simulink model "CalPrmAndSigMgrForCmptmtTCtrl"  (R2022b)
settings: Solver=FixedStepDiscrete  StartTime=0.0  StopTime=10.0  FixedStep=1  RelTol=1e-3
settings Model: Creator=blue.ge  LastModifiedBy=blue.ge
settings DataIOCC: SaveFormat=StructureWithTime
settings OptimizationCC: DefaultParameterBehavior=Inlined  EnhancedBackFolding=on  PassReuseOutputArgsAs=Individual arguments  ZeroExternalMemoryAtStartup=off  ZeroInternalMemoryAtStartup=off  OptimizeBlockOrder=speed  DifferentSizesBufferReuse=on  OptimizationCustomize=off  EfficientTunableParamExpr=on
settings DebuggingCC: ParameterTunabilityLossMsg=error
settings ModelReferenceCC: ModelReferenceNumInstancesAllowed=Single
settings SFSimCC: MATLABDynamicMemAlloc=off
settings RTWCC: Description=AUTOSAR  SystemTargetFile=autosar.tlc  GenCodeOnly=on  TemplateMakefile=ert_default_tmf
settings CodeAppCC: ForceParamTrailComments=on  IgnoreCustomStorageClasses=off  OperatorAnnotations=on  SimulinkDataObjDesc=on  SFDataObjDesc=on  InsertBlockDesc=on
settings STFCustomTargetCC: TargetLangStandard=C99 (ISO)  MultiwordLength=256  ParMdlRefBuildCompliant=on  CompOptLevelCompliant=on  IncludeMdlTerminateFcn=off  CombineOutputUpdateFcns=on  SuppressErrorStatus=on  ERTCustomFileBanners=on  SupportNonFinite=off  SupportComplex=off  SupportContinuousTime=off  SupportNonInlinedSFcns=off  RemoveResetFunc=on  MATLABClassNameForMDSCustomization=Simulink.SoftwareTarget.ERTCustomization  AutosarCompliant=on  UseToolchainInfoCompliant=on  LookupTableObjectStructAxisOrder=2,1,3,4,...  LUTObjectStructOrderEvenSpacing=Size,Table,Breakpoints  SystemTargetFile=autosar.tlc
settings ERTTargetCC: TargetLangStandard=C99 (ISO)  ConcurrentExecutionCompliant=off  IncludeMdlTerminateFcn=off  SuppressErrorStatus=on  SupportNonFinite=off  SupportComplex=off  ModelStepFunctionPrototypeControlCompliant=off  CPPClassGenCompliant=off  AutosarCompliant=on  LookupTableObjectStructAxisOrder=2,1,3,4,...  LUTObjectStructOrderEvenSpacing=Size,Table,Breakpoints  InlinedParameterPlacement=NonHierarchical  ERTCustomFileTemplate=example_file_process.tlc

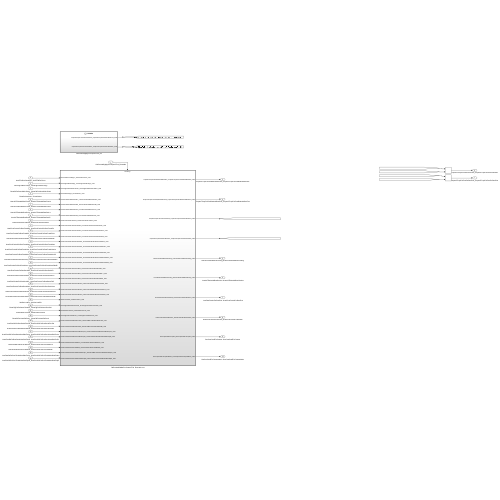
[thumbnail: Simulink's stored preview of the top-level diagram]
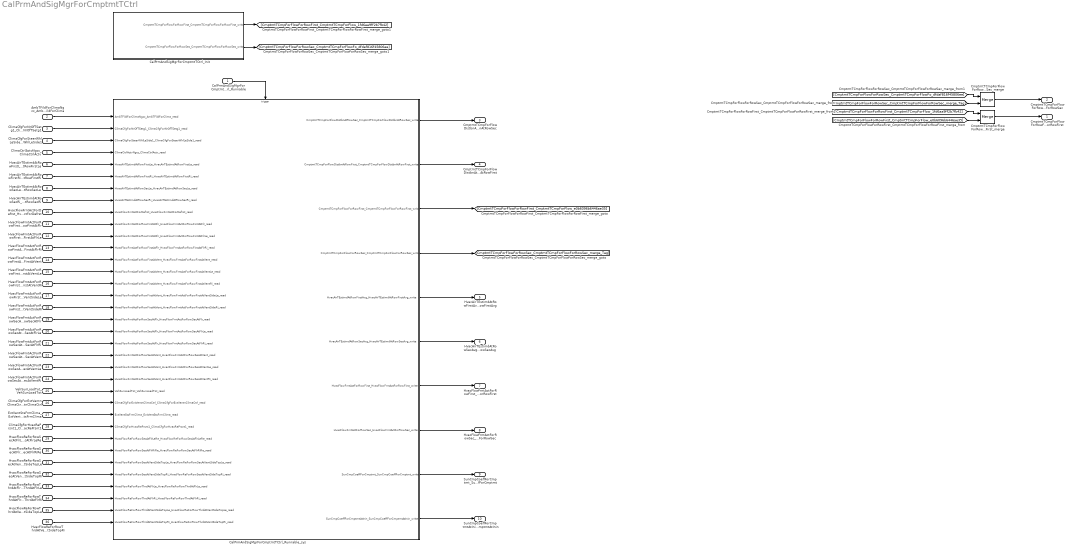
== system CalPrmAndSigMgrForCmptmtTCtrl ==
  CalPrmAndSigMgrForCmptmtTCtrl_Runnable : Inport  SampleTime=1  OutputFunctionCall=on
  AmbTFildForClimaNgcc_AmbTFildForClima : Inport  Port=2  OutDataTypeStr=T30  PortDimensions=1
  ClimaCfgForNrOfTSetg1_ClimaCfgForNrOfTSetg1 : Inport  Port=3  OutDataTypeStr=Enum: ClimaCfgForNrOfTSetg1  PortDimensions=1
  ClimaCfgForSteerWhlLeSide1_ClimaCfgForSteerWhlLeSide1 : Inport  Port=4  OutDataTypeStr=Boolean  PortDimensions=1
  ClimaCtrlActvNgcc_ClimaCtrlActv : Inport  Port=5  OutDataTypeStr=Boolean  PortDimensions=1
  HvacAirTEstimdAtRowFirstLe_HvacAirTEstimdAtRowFirstLe : Inport  Port=6  OutDataTypeStr=T30  PortDimensions=1
  HvacAirTEstimdAtRowFirstRi_HvacAirTEstimdAtRowFirstRi : Inport  Port=7  OutDataTypeStr=T30  PortDimensions=1
  HvacAirTEstimdAtRowSecLe_HvacAirTEstimdAtRowSecLe : Inport  Port=8  OutDataTypeStr=T30  PortDimensions=1
  HvacAirTEstimdAtRowSecRi_HvacAirTEstimdAtRowSecRi : Inport  Port=9  OutDataTypeStr=T30  PortDimensions=1
  HvacFlowFrntActForDefrst_HvacFlowFrntActForDefrst : Inport  Port=10  OutDataTypeStr=AirFlow30  PortDimensions=1
  HvacFlowFrntActForRowFirstAtFlr_HvacFlowFrntActForRowFirstAtFlr : Inport  Port=11  OutDataTypeStr=AirFlow30  PortDimensions=1
  HvacFlowFrntActForRowFirstAtFlr_HvacFlowFrntActForRowFirstAtFlrLe : Inport  Port=12  OutDataTypeStr=AirFlow30  PortDimensions=1
  HvacFlowFrntActForRowFirstAtFlr_HvacFlowFrntActForRowFirstAtFlrRi : Inport  Port=13  OutDataTypeStr=AirFlow30  PortDimensions=1
  HvacFlowFrntActForRowFirstAtVent_HvacFlowFrntActForRowFirstAtVent : Inport  Port=14  OutDataTypeStr=AirFlow30  PortDimensions=1
  HvacFlowFrntActForRowFirstAtVent_HvacFlowFrntActForRowFirstAtVentLe : Inport  Port=15  OutDataTypeStr=AirFlow30  PortDimensions=1
  HvacFlowFrntActForRowFirstAtVent_HvacFlowFrntActForRowFirstAtVentRi : Inport  Port=16  OutDataTypeStr=AirFlow30  PortDimensions=1
  HvacFlowFrntActForRowFirstAtVent_HvacFlowFrntActForRowFirstAtVentSideLe : Inport  Port=17  OutDataTypeStr=AirFlow30  PortDimensions=1
  HvacFlowFrntActForRowFirstAtVent_HvacFlowFrntActForRowFirstAtVentSideRi : Inport  Port=18  OutDataTypeStr=AirFlow30  PortDimensions=1
  HvacFlowFrntActForRowSecAtFlr_HvacFlowFrntActForRowSecAtFlr : Inport  Port=19  OutDataTypeStr=AirFlow30  PortDimensions=1
  HvacFlowFrntActForRowSecAtFlr_HvacFlowFrntActForRowSecAtFlrLe : Inport  Port=20  OutDataTypeStr=AirFlow30  PortDimensions=1
  HvacFlowFrntActForRowSecAtFlr_HvacFlowFrntActForRowSecAtFlrRi : Inport  Port=21  OutDataTypeStr=AirFlow30  PortDimensions=1
  HvacFlowFrntActForRowSecAtVent_HvacFlowFrntActForRowSecAtVent : Inport  Port=22  OutDataTypeStr=AirFlow30  PortDimensions=1
  HvacFlowFrntActForRowSecAtVent_HvacFlowFrntActForRowSecAtVentLe : Inport  Port=23  OutDataTypeStr=AirFlow30  PortDimensions=1
  HvacFlowFrntActForRowSecAtVent_HvacFlowFrntActForRowSecAtVentRi : Inport  Port=24  OutDataTypeStr=AirFlow30  PortDimensions=1
  VehSunLoadTot_VehSunLoadTot : Inport  Port=25  OutDataTypeStr=SunLoad30  PortDimensions=1
  ClimaCfgForExtVentnClimaCtrl_ClimaCfgForExtVentnClimaCtrl : Inport  Port=26  OutDataTypeStr=Enum: CarCfgForExtVentnClimaCtrl  PortDimensions=1
  ExtVentStsFrmClima_ExtVentStsFrmClima : Inport  Port=27  OutDataTypeStr=Enum: OnOff1  PortDimensions=1
  ClimaCfgForHvacRePrsnt1_ClimaCfgForHvacRePrsnt1 : Inport  Port=28  OutDataTypeStr=Enum: CarCfgForHvacRe  PortDimensions=1
  HvacFlowReForRowSecAtFlrLeRe_HvacFlowReForRowSecAtFlrLeRe : Inport  Port=29  OutDataTypeStr=AirFlow30  PortDimensions=1
  HvacFlowReForRowSecAtFlrRiRe_HvacFlowReForRowSecAtFlrRiRe : Inport  Port=30  OutDataTypeStr=AirFlow30  PortDimensions=1
  HvacFlowReForRowSecAtVentSideTopLe_HvacFlowReForRowSecAtVentSideTopLe : Inport  Port=31  OutDataTypeStr=AirFlow30  PortDimensions=1
  HvacFlowReForRowSecAtVentSideTopRi_HvacFlowReForRowSecAtVentSideTopRi : Inport  Port=32  OutDataTypeStr=AirFlow30  PortDimensions=1
  HvacFlowReForRowThrdAtFlrLe_HvacFlowReForRowThrdAtFlrLe : Inport  Port=33  OutDataTypeStr=AirFlow30  PortDimensions=1
  HvacFlowReForRowThrdAtFlrRi_HvacFlowReForRowThrdAtFlrRi : Inport  Port=34  OutDataTypeStr=AirFlow30  PortDimensions=1
  HvacFlowReForRowThrdAtVentSideTopLe_HvacFlowReForRowThrdAtVentSideTopLe : Inport  Port=35  OutDataTypeStr=AirFlow30  PortDimensions=1
  HvacFlowReForRowThrdAtVentSideTopRi_HvacFlowReForRowThrdAtVentSideTopRi : Inport  Port=36  OutDataTypeStr=AirFlow30  PortDimensions=1
  CalPrmAndSigMgrForCmptmtTCtrl_Init : Subsystem
  CalPrmAndSigMgrForCmptmtTCtrl_Runnable_sys : Subsystem
  CmptmtTCmpForFlowForRowFirst_CmptmtTCmpForFlowForRowFirst_merge : Merge
  CmptmtTCmpForFlowForRowSec_CmptmtTCmpForFlowForRowSec_merge : Merge
  CmptmtTCmpForFlowForRowFirst_CmptmtTCmpForFlowForRowFirst : Outport  OutDataTypeStr=Fac30  PortDimensions=1
  CmptmtTCmpForFlowForRowSec_CmptmtTCmpForFlowForRowSec : Outport  Port=2  OutDataTypeStr=Fac30  PortDimensions=1
  CmptmtTCmpForFlowDistbnAtRowSec_CmptmtTCmpForFlowDistbnAtRowSec : Outport  Port=3  OutDataTypeStr=Fac30  PortDimensions=1
  CmptmtTCmpForFlowDistbnAtRowFirst_CmptmtTCmpForFlowDistbnAtRowFirst : Outport  Port=4  OutDataTypeStr=Fac30  PortDimensions=1
  HvacAirTEstimdAtRowFirstAvg_HvacAirTEstimdAtRowFirstAvg : Outport  Port=5  OutDataTypeStr=T30  PortDimensions=1
  HvacAirTEstimdAtRowSecAvg_HvacAirTEstimdAtRowSecAvg : Outport  Port=6  OutDataTypeStr=T30  PortDimensions=1
  HvacFlowFrntActForRowFirst_HvacFlowFrntActForRowFirst : Outport  Port=7  OutDataTypeStr=AirFlow30  PortDimensions=1
  HvacFlowFrntActForRowSec_HvacFlowFrntActForRowSec : Outport  Port=8  OutDataTypeStr=AirFlow30  PortDimensions=1
  SunCmpCoeffForCmptmt_SunCmpCoeffForCmptmt : Outport  Port=9  OutDataTypeStr=Fac30  PortDimensions=1
  SunCmpCoeffForCmptmtAtInin_SunCmpCoeffForCmptmtAtInin : Outport  Port=10  OutDataTypeStr=FacForSunCmpInin  PortDimensions=1
  wires:
  AmbTFildForClimaNgcc_AmbTFildForClima/1 -> CalPrmAndSigMgrForCmptmtTCtrl_Runnable_sys/1(AmbTFildForClimaNgcc_AmbTFildForClima_read)
  ClimaCfgForNrOfTSetg1_ClimaCfgForNrOfTSetg1/1 -> CalPrmAndSigMgrForCmptmtTCtrl_Runnable_sys/2(ClimaCfgForNrOfTSetg1_ClimaCfgForNrOfTSetg1_read)
  ClimaCfgForSteerWhlLeSide1_ClimaCfgForSteerWhlLeSide1/1 -> CalPrmAndSigMgrForCmptmtTCtrl_Runnable_sys/3(ClimaCfgForSteerWhlLeSide1_ClimaCfgForSteerWhlLeSide1_read)
  ClimaCtrlActvNgcc_ClimaCtrlActv/1 -> CalPrmAndSigMgrForCmptmtTCtrl_Runnable_sys/4(ClimaCtrlActvNgcc_ClimaCtrlActv_read)
  HvacAirTEstimdAtRowFirstLe_HvacAirTEstimdAtRowFirstLe/1 -> CalPrmAndSigMgrForCmptmtTCtrl_Runnable_sys/5(HvacAirTEstimdAtRowFirstLe_HvacAirTEstimdAtRowFirstLe_read)
  HvacAirTEstimdAtRowFirstRi_HvacAirTEstimdAtRowFirstRi/1 -> CalPrmAndSigMgrForCmptmtTCtrl_Runnable_sys/6(HvacAirTEstimdAtRowFirstRi_HvacAirTEstimdAtRowFirstRi_read)
  HvacAirTEstimdAtRowSecLe_HvacAirTEstimdAtRowSecLe/1 -> CalPrmAndSigMgrForCmptmtTCtrl_Runnable_sys/7(HvacAirTEstimdAtRowSecLe_HvacAirTEstimdAtRowSecLe_read)
  HvacAirTEstimdAtRowSecRi_HvacAirTEstimdAtRowSecRi/1 -> CalPrmAndSigMgrForCmptmtTCtrl_Runnable_sys/8(HvacAirTEstimdAtRowSecRi_HvacAirTEstimdAtRowSecRi_read)
  HvacFlowFrntActForDefrst_HvacFlowFrntActForDefrst/1 -> CalPrmAndSigMgrForCmptmtTCtrl_Runnable_sys/9(HvacFlowFrntActForDefrst_HvacFlowFrntActForDefrst_read)
  HvacFlowFrntActForRowFirstAtFlr_HvacFlowFrntActForRowFirstAtFlr/1 -> CalPrmAndSigMgrForCmptmtTCtrl_Runnable_sys/10(HvacFlowFrntActForRowFirstAtFlr_HvacFlowFrntActForRowFirstAtFlr_read)
  HvacFlowFrntActForRowFirstAtFlr_HvacFlowFrntActForRowFirstAtFlrLe/1 -> CalPrmAndSigMgrForCmptmtTCtrl_Runnable_sys/11(HvacFlowFrntActForRowFirstAtFlr_HvacFlowFrntActForRowFirstAtFlrLe_read)
  HvacFlowFrntActForRowFirstAtFlr_HvacFlowFrntActForRowFirstAtFlrRi/1 -> CalPrmAndSigMgrForCmptmtTCtrl_Runnable_sys/12(HvacFlowFrntActForRowFirstAtFlr_HvacFlowFrntActForRowFirstAtFlrRi_read)
  HvacFlowFrntActForRowFirstAtVent_HvacFlowFrntActForRowFirstAtVent/1 -> CalPrmAndSigMgrForCmptmtTCtrl_Runnable_sys/13(HvacFlowFrntActForRowFirstAtVent_HvacFlowFrntActForRowFirstAtVent_read)
  HvacFlowFrntActForRowFirstAtVent_HvacFlowFrntActForRowFirstAtVentLe/1 -> CalPrmAndSigMgrForCmptmtTCtrl_Runnable_sys/14(HvacFlowFrntActForRowFirstAtVent_HvacFlowFrntActForRowFirstAtVentLe_read)
  HvacFlowFrntActForRowFirstAtVent_HvacFlowFrntActForRowFirstAtVentRi/1 -> CalPrmAndSigMgrForCmptmtTCtrl_Runnable_sys/15(HvacFlowFrntActForRowFirstAtVent_HvacFlowFrntActForRowFirstAtVentRi_read)
  HvacFlowFrntActForRowFirstAtVent_HvacFlowFrntActForRowFirstAtVentSideLe/1 -> CalPrmAndSigMgrForCmptmtTCtrl_Runnable_sys/16(HvacFlowFrntActForRowFirstAtVent_HvacFlowFrntActForRowFirstAtVentSideLe_read)
  HvacFlowFrntActForRowFirstAtVent_HvacFlowFrntActForRowFirstAtVentSideRi/1 -> CalPrmAndSigMgrForCmptmtTCtrl_Runnable_sys/17(HvacFlowFrntActForRowFirstAtVent_HvacFlowFrntActForRowFirstAtVentSideRi_read)
  HvacFlowFrntActForRowSecAtFlr_HvacFlowFrntActForRowSecAtFlr/1 -> CalPrmAndSigMgrForCmptmtTCtrl_Runnable_sys/18(HvacFlowFrntActForRowSecAtFlr_HvacFlowFrntActForRowSecAtFlr_read)
  HvacFlowFrntActForRowSecAtFlr_HvacFlowFrntActForRowSecAtFlrLe/1 -> CalPrmAndSigMgrForCmptmtTCtrl_Runnable_sys/19(HvacFlowFrntActForRowSecAtFlr_HvacFlowFrntActForRowSecAtFlrLe_read)
  HvacFlowFrntActForRowSecAtFlr_HvacFlowFrntActForRowSecAtFlrRi/1 -> CalPrmAndSigMgrForCmptmtTCtrl_Runnable_sys/20(HvacFlowFrntActForRowSecAtFlr_HvacFlowFrntActForRowSecAtFlrRi_read)
  HvacFlowFrntActForRowSecAtVent_HvacFlowFrntActForRowSecAtVent/1 -> CalPrmAndSigMgrForCmptmtTCtrl_Runnable_sys/21(HvacFlowFrntActForRowSecAtVent_HvacFlowFrntActForRowSecAtVent_read)
  HvacFlowFrntActForRowSecAtVent_HvacFlowFrntActForRowSecAtVentLe/1 -> CalPrmAndSigMgrForCmptmtTCtrl_Runnable_sys/22(HvacFlowFrntActForRowSecAtVent_HvacFlowFrntActForRowSecAtVentLe_read)
  HvacFlowFrntActForRowSecAtVent_HvacFlowFrntActForRowSecAtVentRi/1 -> CalPrmAndSigMgrForCmptmtTCtrl_Runnable_sys/23(HvacFlowFrntActForRowSecAtVent_HvacFlowFrntActForRowSecAtVentRi_read)
  VehSunLoadTot_VehSunLoadTot/1 -> CalPrmAndSigMgrForCmptmtTCtrl_Runnable_sys/24(VehSunLoadTot_VehSunLoadTot_read)
  ClimaCfgForExtVentnClimaCtrl_ClimaCfgForExtVentnClimaCtrl/1 -> CalPrmAndSigMgrForCmptmtTCtrl_Runnable_sys/25(ClimaCfgForExtVentnClimaCtrl_ClimaCfgForExtVentnClimaCtrl_read)
  ExtVentStsFrmClima_ExtVentStsFrmClima/1 -> CalPrmAndSigMgrForCmptmtTCtrl_Runnable_sys/26(ExtVentStsFrmClima_ExtVentStsFrmClima_read)
  ClimaCfgForHvacRePrsnt1_ClimaCfgForHvacRePrsnt1/1 -> CalPrmAndSigMgrForCmptmtTCtrl_Runnable_sys/27(ClimaCfgForHvacRePrsnt1_ClimaCfgForHvacRePrsnt1_read)
  HvacFlowReForRowSecAtFlrLeRe_HvacFlowReForRowSecAtFlrLeRe/1 -> CalPrmAndSigMgrForCmptmtTCtrl_Runnable_sys/28(HvacFlowReForRowSecAtFlrLeRe_HvacFlowReForRowSecAtFlrLeRe_read)
  HvacFlowReForRowSecAtFlrRiRe_HvacFlowReForRowSecAtFlrRiRe/1 -> CalPrmAndSigMgrForCmptmtTCtrl_Runnable_sys/29(HvacFlowReForRowSecAtFlrRiRe_HvacFlowReForRowSecAtFlrRiRe_read)
  HvacFlowReForRowSecAtVentSideTopLe_HvacFlowReForRowSecAtVentSideTopLe/1 -> CalPrmAndSigMgrForCmptmtTCtrl_Runnable_sys/30(HvacFlowReForRowSecAtVentSideTopLe_HvacFlowReForRowSecAtVentSideTopLe_read)
  HvacFlowReForRowSecAtVentSideTopRi_HvacFlowReForRowSecAtVentSideTopRi/1 -> CalPrmAndSigMgrForCmptmtTCtrl_Runnable_sys/31(HvacFlowReForRowSecAtVentSideTopRi_HvacFlowReForRowSecAtVentSideTopRi_read)
  HvacFlowReForRowThrdAtFlrLe_HvacFlowReForRowThrdAtFlrLe/1 -> CalPrmAndSigMgrForCmptmtTCtrl_Runnable_sys/32(HvacFlowReForRowThrdAtFlrLe_HvacFlowReForRowThrdAtFlrLe_read)
  HvacFlowReForRowThrdAtFlrRi_HvacFlowReForRowThrdAtFlrRi/1 -> CalPrmAndSigMgrForCmptmtTCtrl_Runnable_sys/33(HvacFlowReForRowThrdAtFlrRi_HvacFlowReForRowThrdAtFlrRi_read)
  HvacFlowReForRowThrdAtVentSideTopLe_HvacFlowReForRowThrdAtVentSideTopLe/1 -> CalPrmAndSigMgrForCmptmtTCtrl_Runnable_sys/34(HvacFlowReForRowThrdAtVentSideTopLe_HvacFlowReForRowThrdAtVentSideTopLe_read)
  HvacFlowReForRowThrdAtVentSideTopRi_HvacFlowReForRowThrdAtVentSideTopRi/1 -> CalPrmAndSigMgrForCmptmtTCtrl_Runnable_sys/35(HvacFlowReForRowThrdAtVentSideTopRi_HvacFlowReForRowThrdAtVentSideTopRi_read)
  CalPrmAndSigMgrForCmptmtTCtrl_Runnable_sys/1(CmptmtTCmpForFlowDistbnAtRowSec_CmptmtTCmpForFlowDistbnAtRowSec_write) -> CmptmtTCmpForFlowDistbnAtRowSec_CmptmtTCmpForFlowDistbnAtRowSec/1
  CalPrmAndSigMgrForCmptmtTCtrl_Runnable_sys/2(CmptmtTCmpForFlowDistbnAtRowFirst_CmptmtTCmpForFlowDistbnAtRowFirst_write) -> CmptmtTCmpForFlowDistbnAtRowFirst_CmptmtTCmpForFlowDistbnAtRowFirst/1
  CmptmtTCmpForFlowForRowFirst_CmptmtTCmpForFlowForRowFirst_merge/1 -> CmptmtTCmpForFlowForRowFirst_CmptmtTCmpForFlowForRowFirst/1
  CmptmtTCmpForFlowForRowSec_CmptmtTCmpForFlowForRowSec_merge/1 -> CmptmtTCmpForFlowForRowSec_CmptmtTCmpForFlowForRowSec/1
  CalPrmAndSigMgrForCmptmtTCtrl_Runnable_sys/5(HvacAirTEstimdAtRowFirstAvg_HvacAirTEstimdAtRowFirstAvg_write) -> HvacAirTEstimdAtRowFirstAvg_HvacAirTEstimdAtRowFirstAvg/1
  CalPrmAndSigMgrForCmptmtTCtrl_Runnable_sys/6(HvacAirTEstimdAtRowSecAvg_HvacAirTEstimdAtRowSecAvg_write) -> HvacAirTEstimdAtRowSecAvg_HvacAirTEstimdAtRowSecAvg/1
  CalPrmAndSigMgrForCmptmtTCtrl_Runnable_sys/7(HvacFlowFrntActForRowFirst_HvacFlowFrntActForRowFirst_write) -> HvacFlowFrntActForRowFirst_HvacFlowFrntActForRowFirst/1
  CalPrmAndSigMgrForCmptmtTCtrl_Runnable_sys/8(HvacFlowFrntActForRowSec_HvacFlowFrntActForRowSec_write) -> HvacFlowFrntActForRowSec_HvacFlowFrntActForRowSec/1
  CalPrmAndSigMgrForCmptmtTCtrl_Runnable_sys/9(SunCmpCoeffForCmptmt_SunCmpCoeffForCmptmt_write) -> SunCmpCoeffForCmptmt_SunCmpCoeffForCmptmt/1
  CalPrmAndSigMgrForCmptmtTCtrl_Runnable_sys/10(SunCmpCoeffForCmptmtAtInin_SunCmpCoeffForCmptmtAtInin_write) -> SunCmpCoeffForCmptmtAtInin_SunCmpCoeffForCmptmtAtInin/1
  CalPrmAndSigMgrForCmptmtTCtrl_Runnable_sys/4(CmptmtTCmpForFlowForRowSec_CmptmtTCmpForFlowForRowSec_write) -> [CmptmtTCmpForFlowForRowSec_CmptmtTCmpForFlowForRowSec_merge_Tag]
  [CmptmtTCmpForFlowForRowSec_CmptmtTCmpForFlowForRowSec_merge_Tag] -> CmptmtTCmpForFlowForRowSec_CmptmtTCmpForFlowForRowSec_merge/2
  CalPrmAndSigMgrForCmptmtTCtrl_Init/2(CmptmtTCmpForFlowForRowSec_CmptmtTCmpForFlowForRowSec_write) -> [CmptmtTCmpForFlowForRowSec_CmptmtTCmpForFlowFo_dfdaf816f45806ee]
  [CmptmtTCmpForFlowForRowSec_CmptmtTCmpForFlowFo_dfdaf816f45806ee] -> CmptmtTCmpForFlowForRowSec_CmptmtTCmpForFlowForRowSec_merge/1
  CalPrmAndSigMgrForCmptmtTCtrl_Runnable_sys/3(CmptmtTCmpForFlowForRowFirst_CmptmtTCmpForFlowForRowFirst_write) -> [CmptmtTCmpForFlowForRowFirst_CmptmtTCmpForFlow_e0b6096b6446ae35]
  [CmptmtTCmpForFlowForRowFirst_CmptmtTCmpForFlow_e0b6096b6446ae35] -> CmptmtTCmpForFlowForRowFirst_CmptmtTCmpForFlowForRowFirst_merge/2
  CalPrmAndSigMgrForCmptmtTCtrl_Init/1(CmptmtTCmpForFlowForRowFirst_CmptmtTCmpForFlowForRowFirst_write) -> [CmptmtTCmpForFlowForRowFirst_CmptmtTCmpForFlow_1fd6aa9ff2b7fb42]
  [CmptmtTCmpForFlowForRowFirst_CmptmtTCmpForFlow_1fd6aa9ff2b7fb42] -> CmptmtTCmpForFlowForRowFirst_CmptmtTCmpForFlowForRowFirst_merge/1
  CalPrmAndSigMgrForCmptmtTCtrl_Runnable/1 -> CalPrmAndSigMgrForCmptmtTCtrl_Runnable_sys/Trigger

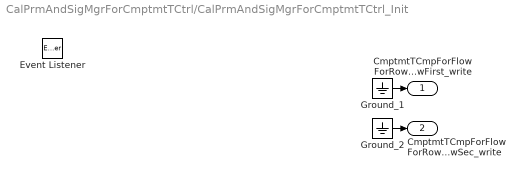
== system CalPrmAndSigMgrForCmptmtTCtrl/CalPrmAndSigMgrForCmptmtTCtrl_Init ==
  Event Listener : EventListener  EventName=reset
  Ground_1 : Ground
  Ground_2 : Ground
  CmptmtTCmpForFlowForRowFirst_CmptmtTCmpForFlowForRowFirst_write : Outport
  CmptmtTCmpForFlowForRowSec_CmptmtTCmpForFlowForRowSec_write : Outport  Port=2
  wires:
  Ground_1/1 -> CmptmtTCmpForFlowForRowFirst_CmptmtTCmpForFlowForRowFirst_write/1
  Ground_2/1 -> CmptmtTCmpForFlowForRowSec_CmptmtTCmpForFlowForRowSec_write/1

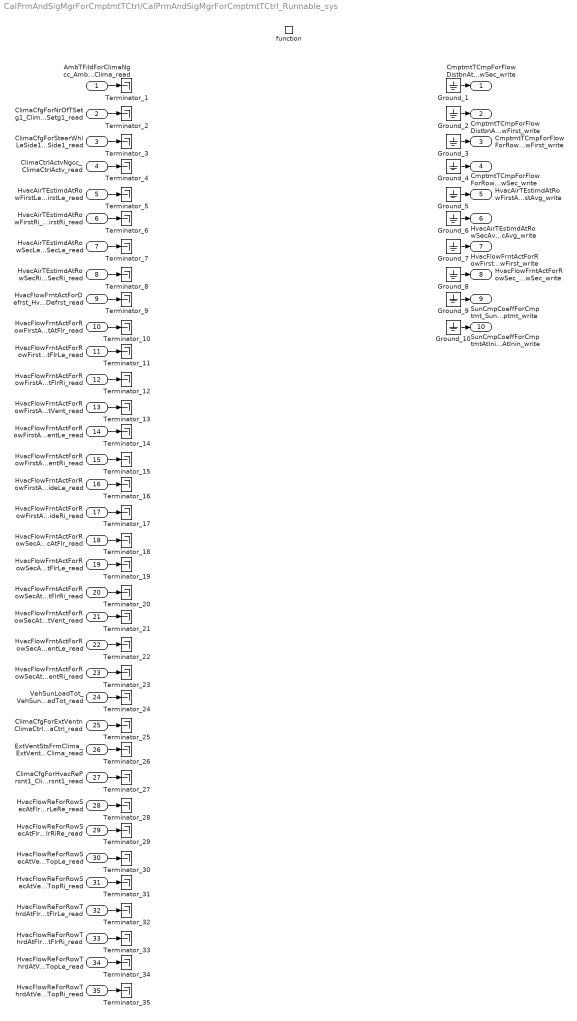
== system CalPrmAndSigMgrForCmptmtTCtrl/CalPrmAndSigMgrForCmptmtTCtrl_Runnable_sys ==
  AmbTFildForClimaNgcc_AmbTFildForClima_read : Inport
  ClimaCfgForNrOfTSetg1_ClimaCfgForNrOfTSetg1_read : Inport  Port=2
  ClimaCfgForSteerWhlLeSide1_ClimaCfgForSteerWhlLeSide1_read : Inport  Port=3
  ClimaCtrlActvNgcc_ClimaCtrlActv_read : Inport  Port=4
  HvacAirTEstimdAtRowFirstLe_HvacAirTEstimdAtRowFirstLe_read : Inport  Port=5
  HvacAirTEstimdAtRowFirstRi_HvacAirTEstimdAtRowFirstRi_read : Inport  Port=6
  HvacAirTEstimdAtRowSecLe_HvacAirTEstimdAtRowSecLe_read : Inport  Port=7
  HvacAirTEstimdAtRowSecRi_HvacAirTEstimdAtRowSecRi_read : Inport  Port=8
  HvacFlowFrntActForDefrst_HvacFlowFrntActForDefrst_read : Inport  Port=9
  HvacFlowFrntActForRowFirstAtFlr_HvacFlowFrntActForRowFirstAtFlr_read : Inport  Port=10
  HvacFlowFrntActForRowFirstAtFlr_HvacFlowFrntActForRowFirstAtFlrLe_read : Inport  Port=11
  HvacFlowFrntActForRowFirstAtFlr_HvacFlowFrntActForRowFirstAtFlrRi_read : Inport  Port=12
  HvacFlowFrntActForRowFirstAtVent_HvacFlowFrntActForRowFirstAtVent_read : Inport  Port=13
  HvacFlowFrntActForRowFirstAtVent_HvacFlowFrntActForRowFirstAtVentLe_read : Inport  Port=14
  HvacFlowFrntActForRowFirstAtVent_HvacFlowFrntActForRowFirstAtVentRi_read : Inport  Port=15
  HvacFlowFrntActForRowFirstAtVent_HvacFlowFrntActForRowFirstAtVentSideLe_read : Inport  Port=16
  HvacFlowFrntActForRowFirstAtVent_HvacFlowFrntActForRowFirstAtVentSideRi_read : Inport  Port=17
  HvacFlowFrntActForRowSecAtFlr_HvacFlowFrntActForRowSecAtFlr_read : Inport  Port=18
  HvacFlowFrntActForRowSecAtFlr_HvacFlowFrntActForRowSecAtFlrLe_read : Inport  Port=19
  HvacFlowFrntActForRowSecAtFlr_HvacFlowFrntActForRowSecAtFlrRi_read : Inport  Port=20
  HvacFlowFrntActForRowSecAtVent_HvacFlowFrntActForRowSecAtVent_read : Inport  Port=21
  HvacFlowFrntActForRowSecAtVent_HvacFlowFrntActForRowSecAtVentLe_read : Inport  Port=22
  HvacFlowFrntActForRowSecAtVent_HvacFlowFrntActForRowSecAtVentRi_read : Inport  Port=23
  VehSunLoadTot_VehSunLoadTot_read : Inport  Port=24
  ClimaCfgForExtVentnClimaCtrl_ClimaCfgForExtVentnClimaCtrl_read : Inport  Port=25
  ExtVentStsFrmClima_ExtVentStsFrmClima_read : Inport  Port=26
  ClimaCfgForHvacRePrsnt1_ClimaCfgForHvacRePrsnt1_read : Inport  Port=27
  HvacFlowReForRowSecAtFlrLeRe_HvacFlowReForRowSecAtFlrLeRe_read : Inport  Port=28
  HvacFlowReForRowSecAtFlrRiRe_HvacFlowReForRowSecAtFlrRiRe_read : Inport  Port=29
  HvacFlowReForRowSecAtVentSideTopLe_HvacFlowReForRowSecAtVentSideTopLe_read : Inport  Port=30
  HvacFlowReForRowSecAtVentSideTopRi_HvacFlowReForRowSecAtVentSideTopRi_read : Inport  Port=31
  HvacFlowReForRowThrdAtFlrLe_HvacFlowReForRowThrdAtFlrLe_read : Inport  Port=32
  HvacFlowReForRowThrdAtFlrRi_HvacFlowReForRowThrdAtFlrRi_read : Inport  Port=33
  HvacFlowReForRowThrdAtVentSideTopLe_HvacFlowReForRowThrdAtVentSideTopLe_read : Inport  Port=34
  HvacFlowReForRowThrdAtVentSideTopRi_HvacFlowReForRowThrdAtVentSideTopRi_read : Inport  Port=35
  function : TriggerPort  TriggerType=function-call  VariantControl=(inherit)  SampleTimeType=periodic  SampleTime=-1
  Ground_{1..10} (10 alike) : Ground
  Terminator_{1..35} (35 alike) : Terminator
  CmptmtTCmpForFlowDistbnAtRowSec_CmptmtTCmpForFlowDistbnAtRowSec_write : Outport
      settings: EnsureOutportIsVirtual=on
  CmptmtTCmpForFlowDistbnAtRowFirst_CmptmtTCmpForFlowDistbnAtRowFirst_write : Outport  Port=2
      settings: EnsureOutportIsVirtual=on
  CmptmtTCmpForFlowForRowFirst_CmptmtTCmpForFlowForRowFirst_write : Outport  Port=3
      settings: EnsureOutportIsVirtual=on
  CmptmtTCmpForFlowForRowSec_CmptmtTCmpForFlowForRowSec_write : Outport  Port=4
      settings: EnsureOutportIsVirtual=on
  HvacAirTEstimdAtRowFirstAvg_HvacAirTEstimdAtRowFirstAvg_write : Outport  Port=5
      settings: EnsureOutportIsVirtual=on
  HvacAirTEstimdAtRowSecAvg_HvacAirTEstimdAtRowSecAvg_write : Outport  Port=6
      settings: EnsureOutportIsVirtual=on
  HvacFlowFrntActForRowFirst_HvacFlowFrntActForRowFirst_write : Outport  Port=7
      settings: EnsureOutportIsVirtual=on
  HvacFlowFrntActForRowSec_HvacFlowFrntActForRowSec_write : Outport  Port=8
      settings: EnsureOutportIsVirtual=on
  SunCmpCoeffForCmptmt_SunCmpCoeffForCmptmt_write : Outport  Port=9
      settings: EnsureOutportIsVirtual=on
  SunCmpCoeffForCmptmtAtInin_SunCmpCoeffForCmptmtAtInin_write : Outport  Port=10
      settings: EnsureOutportIsVirtual=on
  wires:
  AmbTFildForClimaNgcc_AmbTFildForClima_read/1 -> Terminator_1/1
  ClimaCfgForNrOfTSetg1_ClimaCfgForNrOfTSetg1_read/1 -> Terminator_2/1
  ClimaCfgForSteerWhlLeSide1_ClimaCfgForSteerWhlLeSide1_read/1 -> Terminator_3/1
  ClimaCtrlActvNgcc_ClimaCtrlActv_read/1 -> Terminator_4/1
  HvacAirTEstimdAtRowFirstLe_HvacAirTEstimdAtRowFirstLe_read/1 -> Terminator_5/1
  HvacAirTEstimdAtRowFirstRi_HvacAirTEstimdAtRowFirstRi_read/1 -> Terminator_6/1
  HvacAirTEstimdAtRowSecLe_HvacAirTEstimdAtRowSecLe_read/1 -> Terminator_7/1
  HvacAirTEstimdAtRowSecRi_HvacAirTEstimdAtRowSecRi_read/1 -> Terminator_8/1
  HvacFlowFrntActForDefrst_HvacFlowFrntActForDefrst_read/1 -> Terminator_9/1
  HvacFlowFrntActForRowFirstAtFlr_HvacFlowFrntActForRowFirstAtFlr_read/1 -> Terminator_10/1
  HvacFlowFrntActForRowFirstAtFlr_HvacFlowFrntActForRowFirstAtFlrLe_read/1 -> Terminator_11/1
  HvacFlowFrntActForRowFirstAtFlr_HvacFlowFrntActForRowFirstAtFlrRi_read/1 -> Terminator_12/1
  HvacFlowFrntActForRowFirstAtVent_HvacFlowFrntActForRowFirstAtVent_read/1 -> Terminator_13/1
  HvacFlowFrntActForRowFirstAtVent_HvacFlowFrntActForRowFirstAtVentLe_read/1 -> Terminator_14/1
  HvacFlowFrntActForRowFirstAtVent_HvacFlowFrntActForRowFirstAtVentRi_read/1 -> Terminator_15/1
  HvacFlowFrntActForRowFirstAtVent_HvacFlowFrntActForRowFirstAtVentSideLe_read/1 -> Terminator_16/1
  HvacFlowFrntActForRowFirstAtVent_HvacFlowFrntActForRowFirstAtVentSideRi_read/1 -> Terminator_17/1
  HvacFlowFrntActForRowSecAtFlr_HvacFlowFrntActForRowSecAtFlr_read/1 -> Terminator_18/1
  HvacFlowFrntActForRowSecAtFlr_HvacFlowFrntActForRowSecAtFlrLe_read/1 -> Terminator_19/1
  HvacFlowFrntActForRowSecAtFlr_HvacFlowFrntActForRowSecAtFlrRi_read/1 -> Terminator_20/1
  HvacFlowFrntActForRowSecAtVent_HvacFlowFrntActForRowSecAtVent_read/1 -> Terminator_21/1
  HvacFlowFrntActForRowSecAtVent_HvacFlowFrntActForRowSecAtVentLe_read/1 -> Terminator_22/1
  HvacFlowFrntActForRowSecAtVent_HvacFlowFrntActForRowSecAtVentRi_read/1 -> Terminator_23/1
  VehSunLoadTot_VehSunLoadTot_read/1 -> Terminator_24/1
  ClimaCfgForExtVentnClimaCtrl_ClimaCfgForExtVentnClimaCtrl_read/1 -> Terminator_25/1
  ExtVentStsFrmClima_ExtVentStsFrmClima_read/1 -> Terminator_26/1
  ClimaCfgForHvacRePrsnt1_ClimaCfgForHvacRePrsnt1_read/1 -> Terminator_27/1
  HvacFlowReForRowSecAtFlrLeRe_HvacFlowReForRowSecAtFlrLeRe_read/1 -> Terminator_28/1
  HvacFlowReForRowSecAtFlrRiRe_HvacFlowReForRowSecAtFlrRiRe_read/1 -> Terminator_29/1
  HvacFlowReForRowSecAtVentSideTopLe_HvacFlowReForRowSecAtVentSideTopLe_read/1 -> Terminator_30/1
  HvacFlowReForRowSecAtVentSideTopRi_HvacFlowReForRowSecAtVentSideTopRi_read/1 -> Terminator_31/1
  HvacFlowReForRowThrdAtFlrLe_HvacFlowReForRowThrdAtFlrLe_read/1 -> Terminator_32/1
  HvacFlowReForRowThrdAtFlrRi_HvacFlowReForRowThrdAtFlrRi_read/1 -> Terminator_33/1
  HvacFlowReForRowThrdAtVentSideTopLe_HvacFlowReForRowThrdAtVentSideTopLe_read/1 -> Terminator_34/1
  HvacFlowReForRowThrdAtVentSideTopRi_HvacFlowReForRowThrdAtVentSideTopRi_read/1 -> Terminator_35/1
  Ground_1/1 -> CmptmtTCmpForFlowDistbnAtRowSec_CmptmtTCmpForFlowDistbnAtRowSec_write/1
  Ground_2/1 -> CmptmtTCmpForFlowDistbnAtRowFirst_CmptmtTCmpForFlowDistbnAtRowFirst_write/1
  Ground_3/1 -> CmptmtTCmpForFlowForRowFirst_CmptmtTCmpForFlowForRowFirst_write/1
  Ground_4/1 -> CmptmtTCmpForFlowForRowSec_CmptmtTCmpForFlowForRowSec_write/1
  Ground_5/1 -> HvacAirTEstimdAtRowFirstAvg_HvacAirTEstimdAtRowFirstAvg_write/1
  Ground_6/1 -> HvacAirTEstimdAtRowSecAvg_HvacAirTEstimdAtRowSecAvg_write/1
  Ground_7/1 -> HvacFlowFrntActForRowFirst_HvacFlowFrntActForRowFirst_write/1
  Ground_8/1 -> HvacFlowFrntActForRowSec_HvacFlowFrntActForRowSec_write/1
  Ground_9/1 -> SunCmpCoeffForCmptmt_SunCmpCoeffForCmptmt_write/1
  Ground_10/1 -> SunCmpCoeffForCmptmtAtInin_SunCmpCoeffForCmptmtAtInin_write/1
